simulink subsystem "ButtonStatesRF"  (R2023b) (continued)
== stateflow chart ButtonStatesRF/ButtonStates/buttonFlowChart == (continued)
                (junction 6907) -> 6908
                QS_DOWN_SubMenu -> 6908 [order 2] : EXIT
                QS_DOWN_SubMenu -> (junction 6907) [order 1] : SET
                    | {quickSelectorDown = currentState;
                    | BLU_FnSetQuickSelectorDOWN(quickSelectorDown);}
                6909 -> QS_DOWN_SubMenu
              QuickSelectorUP -> 6783 [order 1] : SET
              6784 -> QuickSelectorSubMenu1
              6785 -> QuickSelectorUP
              QuickSelectorSubMenu1 -> 6786 : EXIT
              QuickSelectorDOWN -> 6787 [order 1] : SET
              6788 -> QuickSelectorDOWN
            state ConnectivityMenu
              (history)
              state ConnectivitySubMenu
                (history)
                state ConnectivitySubMenu
                  state Bluetooth
                    en:
                    currentState = eStateName.CONNECTIVITY_BLUETOOTH;
                  state PhonePairing
                    en:
                    currentState = eStateName.CONNECTIVITY_PHONEPAIRING;
                  state HeadsetPairing
                    en:
                    currentState = eStateName.CONNECTIVITY_HEADSETPAIRING;
                  state HeadsetType
                    en:
                    currentState = eStateName.CONNECTIVITY_HEADSETTYPE;
                  (default) -> (junction 7044)
                  (junction 7044) -> Bluetooth
                  (junction 7051) -> (junction 7044)
                  Bluetooth -> (junction 7045) [order 3] : UP
                  (junction 7045) -> (junction 7050) : [CCU_BT_Status]
                  Bluetooth -> (junction 7046) [order 1] : DOWN
                  PhonePairing -> Bluetooth [order 2] : UP
                  (junction 7046) -> PhonePairing : [CCU_BT_Status]
                  (junction 7049) -> (junction 7046) [order 2]
                  PhonePairing -> (junction 7047) [order 1] : DOWN
                  (junction 7047) -> (junction 7048)
                  HeadsetPairing -> PhonePairing [order 1] : UP
                  (junction 7048) -> HeadsetPairing [order 1] : [CCU_Corded_Headset == 0]
                  (junction 7048) -> (junction 7050) [order 2]
                  (junction 7049) -> HeadsetPairing [order 1] : [CCU_Corded_Headset == 0]
                  HeadsetType -> (junction 7049) [order 1] : UP
                  HeadsetPairing -> HeadsetType [order 2] : DOWN
                  (junction 7050) -> HeadsetType
                  HeadsetType -> (junction 7051) [order 3] : DOWN
                state BLUETOOTH_ONOFF
                  (history)
                  state BT_Sel
                    state BLUETOOTH_ON
                      en:
                      currentState = eStateName.CONNECTIVITY_BLUETOOTH_ON;
                    state BLUETOOTH_OFF
                      en:
                      currentState = eStateName.CONNECTIVITY_BLUETOOTH_OFF ;
                    (junction 7084) -> BLUETOOTH_ON [order 1] : [CCU_BT_Status]
                    BLUETOOTH_ON -> BLUETOOTH_OFF : DOWN || UP
                    BLUETOOTH_OFF -> BLUETOOTH_ON : DOWN || UP
                    (default) -> (junction 7084)
                    (junction 7084) -> BLUETOOTH_OFF [order 2]
                  BT_Sel -> (junction 7076) [order 2] : SET
                  (junction 7076) -> 7077 : {BLU_FnConnBTSwitch(currentState);}
                  BT_Sel -> 7077 [order 1] : EXIT
                  7078 -> (junction 7084)
                state PhoneMenu
                  (history)
                  state PhonePairinSubMenu
                    state NEW_PAIRING
                      en:
                      currentState = eStateName.PHONE_PAIRING_NEW;
                    state DELETE_PAIRING
                      en:
                      currentState = eStateName.PHONE_PAIRING_DELETE ;
                    (junction 7112) -> NEW_PAIRING [order 2]
                    NEW_PAIRING -> (junction 7113) [order 2] : DOWN || UP
                    NEW_PAIRING -> DELETE_PAIRING [order 3] : [hasChangedTo(btPhonePrngScs,true)]
                    DELETE_PAIRING -> NEW_PAIRING [order 3] : [hasChangedTo(btPhonePrngScs,false)]
                    (junction 7111) -> (junction 7112)
                    (junction 7113) -> (junction 7112)
                    (default) -> (junction 7111)
                    (junction 7112) -> DELETE_PAIRING [order 1] : [btPhonePrngScs]
                    DELETE_PAIRING -> (junction 7113) [order 1] : DOWN || UP
                  state PhoneDeviceSearch
                    (history)
                    state BT_PHONE_STRT_SEARCHING
                      en:
                      currentState=eStateName.BT_PHONE_STRT_SEARCHING;
                    state BT_PHONE_FOUND
                      en:
                      currentState=eStateName.BT_PHONE_DVC_LIST;
                    state BT_PHONE_ALREADY_PAIRED
                      en:
                      currentState=eStateName.BT_PHONE_ALREADY_PAIRED;
                    state BT_PHONE_NOT_FOUND [commented out]
                      en:
                      currentState=eStateName.BT_PHONE_NOT_FOUND;
                    state BT_PHONE_PAIRING
                      en:
                      currentState=eStateName.BT_PHONE_PAIRING_INPROGRESS;
                    state BT_PHONE_CHECK_PASSKEY
                      en:
                      currentState=eStateName.BT_PHONE_CHECK_PASSKEY;
                    state BT_PHONE_PAIRING_FAILED
                      en:
                      currentState=eStateName.BT_PHONE_PAIRING_FAILED;
                    state BT_PHONE_PAIR_SUCCESS
                      en:
                      currentState=eStateName.BT_PHONE_PAIR_SUCCESS;
                    7146 -> Flow.ButtonTriggeredMenu.Menu.MainSubMenu.SettingsSubMenu.ConnectivityMenu.ConnectivitySubMenu.PhoneMenu.PhoneDeviceSearch.BT_PHONE_STRT_SEARCHING
                    (default) -> Flow.ButtonTriggeredMenu.Menu.MainSubMenu.SettingsSubMenu.ConnectivityMenu.ConnectivitySubMenu.PhoneMenu.PhoneDeviceSearch.BT_PHONE_STRT_SEARCHING
                    Flow.ButtonTriggeredMenu.Menu.MainSubMenu.SettingsSubMenu.ConnectivityMenu.ConnectivitySubMenu.PhoneMenu.PhoneDeviceSearch.BT_PHONE_FOUND -> (junction 7127) [order 1] : UP
                    (junction 7127) -> (junction 7128) : {BLU_FnBTPhoneDvcUp();}
                    Flow.ButtonTriggeredMenu.Menu.MainSubMenu.SettingsSubMenu.ConnectivityMenu.ConnectivitySubMenu.PhoneMenu.PhoneDeviceSearch.BT_PHONE_STRT_SEARCHING -> Flow.ButtonTriggeredMenu.Menu.MainSubMenu.SettingsSubMenu.ConnectivityMenu.ConnectivitySubMenu.PhoneMenu.PhoneDeviceSearch.BT_PHONE_FOUND [order 1] : [hasChangedTo(btPhoneFound,true)]
                    (junction 7128) -> Flow.ButtonTriggeredMenu.Menu.MainSubMenu.SettingsSubMenu.ConnectivityMenu.ConnectivitySubMenu.PhoneMenu.PhoneDeviceSearch.BT_PHONE_FOUND
                    (junction 7129) -> (junction 7128) : {BLU_FnBTPhoneDvcDwn();}
                    Flow.ButtonTriggeredMenu.Menu.MainSubMenu.SettingsSubMenu.ConnectivityMenu.ConnectivitySubMenu.PhoneMenu.PhoneDeviceSearch.BT_PHONE_STRT_SEARCHING -> (junction 7130) [order 3] : after(BLU_BTSearchDur_Tms,msec)
                    Flow.ButtonTriggeredMenu.Menu.MainSubMenu.SettingsSubMenu.ConnectivityMenu.ConnectivitySubMenu.PhoneMenu.PhoneDeviceSearch.BT_PHONE_STRT_SEARCHING -> (junction 7131) [order 2] : EXIT
                    Flow.ButtonTriggeredMenu.Menu.MainSubMenu.SettingsSubMenu.ConnectivityMenu.ConnectivitySubMenu.PhoneMenu.PhoneDeviceSearch.BT_PHONE_FOUND -> (junction 7129) [order 2] : DOWN
                    (junction 7130) -> (junction 7131)
                    (junction 7131) -> (junction 7132)
                    Flow.ButtonTriggeredMenu.Menu.MainSubMenu.SettingsSubMenu.ConnectivityMenu.ConnectivitySubMenu.PhoneMenu.PhoneDeviceSearch.BT_PHONE_FOUND -> (junction 7132) [order 3] : EXIT
                    Flow.ButtonTriggeredMenu.Menu.MainSubMenu.SettingsSubMenu.ConnectivityMenu.ConnectivitySubMenu.PhoneMenu.PhoneDeviceSearch.BT_PHONE_FOUND -> (junction 7133) [order 4] : SET
                    (junction 7132) -> (junction 7135)
                    (junction 7133) -> (junction 7134)
                    (junction 7134) -> Flow.ButtonTriggeredMenu.Menu.MainSubMenu.SettingsSubMenu.ConnectivityMenu.ConnectivitySubMenu.PhoneMenu.PhoneDeviceSearch.BT_PHONE_ALREADY_PAIRED [order 1] : %[phonePaired]
                        | [btPhonePrngScs]
                    (junction 7134) -> (junction 7136) [order 2]
                    Flow.ButtonTriggeredMenu.Menu.MainSubMenu.SettingsSubMenu.ConnectivityMenu.ConnectivitySubMenu.PhoneMenu.PhoneDeviceSearch.BT_PHONE_NOT_FOUND -> (junction 7135) [commented out] : EXIT
                    Flow.ButtonTriggeredMenu.Menu.MainSubMenu.SettingsSubMenu.ConnectivityMenu.ConnectivitySubMenu.PhoneMenu.PhoneDeviceSearch.BT_PHONE_ALREADY_PAIRED -> (junction 7135) [order 2] : EXIT
                    (junction 7135) -> (junction 7140)
                    Flow.ButtonTriggeredMenu.Menu.MainSubMenu.SettingsSubMenu.ConnectivityMenu.ConnectivitySubMenu.PhoneMenu.PhoneDeviceSearch.BT_PHONE_ALREADY_PAIRED -> (junction 7136) [order 1] : SET
                    (junction 7136) -> Flow.ButtonTriggeredMenu.Menu.MainSubMenu.SettingsSubMenu.ConnectivityMenu.ConnectivitySubMenu.PhoneMenu.PhoneDeviceSearch.BT_PHONE_PAIRING : {BLU_FnBTPhonePairReq();}
                    (junction 7138) -> (junction 7137)
                    (junction 7137) -> (junction 7139)
                    Flow.ButtonTriggeredMenu.Menu.MainSubMenu.SettingsSubMenu.ConnectivityMenu.ConnectivitySubMenu.PhoneMenu.PhoneDeviceSearch.BT_PHONE_CHECK_PASSKEY -> (junction 7138) [order 2] : [hasChangedTo(btPhonePairingError,true)]
                    Flow.ButtonTriggeredMenu.Menu.MainSubMenu.SettingsSubMenu.ConnectivityMenu.ConnectivitySubMenu.PhoneMenu.PhoneDeviceSearch.BT_PHONE_CHECK_PASSKEY -> (junction 7139) [order 1] : EXIT
                    Flow.ButtonTriggeredMenu.Menu.MainSubMenu.SettingsSubMenu.ConnectivityMenu.ConnectivitySubMenu.PhoneMenu.PhoneDeviceSearch.BT_PHONE_PAIRING -> Flow.ButtonTriggeredMenu.Menu.MainSubMenu.SettingsSubMenu.ConnectivityMenu.ConnectivitySubMenu.PhoneMenu.PhoneDeviceSearch.BT_PHONE_CHECK_PASSKEY [order 3] : [hasChangedTo(btPhonePassKeyRcvd,true)]
                    (junction 7139) -> Flow.ButtonTriggeredMenu.Menu.MainSubMenu.SettingsSubMenu.ConnectivityMenu.ConnectivitySubMenu.PhoneMenu.PhoneDeviceSearch.BT_PHONE_PAIRING_FAILED
                    Flow.ButtonTriggeredMenu.Menu.MainSubMenu.SettingsSubMenu.ConnectivityMenu.ConnectivitySubMenu.PhoneMenu.PhoneDeviceSearch.BT_PHONE_CHECK_PASSKEY -> (junction 7140) [order 4] : [after(25,sec)]
                    Flow.ButtonTriggeredMenu.Menu.MainSubMenu.SettingsSubMenu.ConnectivityMenu.ConnectivitySubMenu.PhoneMenu.PhoneDeviceSearch.BT_PHONE_CHECK_PASSKEY -> Flow.ButtonTriggeredMenu.Menu.MainSubMenu.SettingsSubMenu.ConnectivityMenu.ConnectivitySubMenu.PhoneMenu.PhoneDeviceSearch.BT_PHONE_PAIRING [order 3] : SET
                        | {BLU_FnBTPhonePassKeyChkd();}
                    (junction 7140) -> (junction 7141)
                    Flow.ButtonTriggeredMenu.Menu.MainSubMenu.SettingsSubMenu.ConnectivityMenu.ConnectivitySubMenu.PhoneMenu.PhoneDeviceSearch.BT_PHONE_PAIRING -> (junction 7142) [order 4] : EXIT
                    Flow.ButtonTriggeredMenu.Menu.MainSubMenu.SettingsSubMenu.ConnectivityMenu.ConnectivitySubMenu.PhoneMenu.PhoneDeviceSearch.BT_PHONE_PAIRING -> (junction 7143) [order 1] : [hasChangedTo(btPhonePairingError,true)]
                    Flow.ButtonTriggeredMenu.Menu.MainSubMenu.SettingsSubMenu.ConnectivityMenu.ConnectivitySubMenu.PhoneMenu.PhoneDeviceSearch.BT_PHONE_PAIRING -> (junction 7144) [order 2] : [hasChangedTo(btPhonePrngScs,true)]
                    Flow.ButtonTriggeredMenu.Menu.MainSubMenu.SettingsSubMenu.ConnectivityMenu.ConnectivitySubMenu.PhoneMenu.PhoneDeviceSearch.BT_PHONE_PAIRING_FAILED -> (junction 7141) : [after(3,sec)]
                        | {phonePaired=false;}
                    (junction 7142) -> (junction 7141)
                    (junction 7143) -> (junction 7142)
                    (junction 7141) -> (junction 7145)
                    (junction 7145) -> 7147
                    Flow.ButtonTriggeredMenu.Menu.MainSubMenu.SettingsSubMenu.ConnectivityMenu.ConnectivitySubMenu.PhoneMenu.PhoneDeviceSearch.BT_PHONE_PAIR_SUCCESS -> (junction 7145) : after(1,sec)
                    (junction 7144) -> Flow.ButtonTriggeredMenu.Menu.MainSubMenu.SettingsSubMenu.ConnectivityMenu.ConnectivitySubMenu.PhoneMenu.PhoneDeviceSearch.BT_PHONE_PAIR_SUCCESS : {phonePaired=true;}
                  state PhoneBTDvcDelete
                    (history)
                    state BT_PHONE_DEL_PAIRING_WAIT
                      en:
                      currentState=eStateName.BT_PHONE_DEL_PAIRING_WAIT;
                      BLU_FnBTPhoneDvcDel();
                    7202 -> (junction 7201)
                    (junction 7200) -> 7203
                    BT_PHONE_DEL_PAIRING_WAIT -> (junction 7200) [order 1] : EXIT
                    BT_PHONE_DEL_PAIRING_WAIT -> 7203 [order 2] : [hasChangedTo(btPhoneDelErr,true)]
                    (default) -> (junction 7201)
                    (junction 7201) -> BT_PHONE_DEL_PAIRING_WAIT
                    BT_PHONE_DEL_PAIRING_WAIT -> 7204 [order 3] : [hasChangedTo(btPhonePrngScs, false)]
                  NEW_PAIRING -> 7095 [order 1] : SET
                  7096 -> PhonePairinSubMenu
                  (junction 7094) -> NEW_PAIRING
                  7098 -> (junction 7094)
                  7097 -> (junction 7111)
                  DELETE_PAIRING -> 7099 [order 2] : SET
                  PhonePairinSubMenu -> 7100 : EXIT
                  7101 -> DELETE_PAIRING
                state BT_HeadsetMenu
                  (history)
                  state HeadsetPairingSubMenu
                    state HEADSET_PAIRING_NEW
                      en:
                      currentState = eStateName.HEADSET_PAIRING_NEW;
                    state HEADSET_PAIRING_DELETE
                      en:
                      currentState = eStateName.HEADSET_PAIRING_DELETE;
                    (junction 7232) -> HEADSET_PAIRING_NEW [order 2]
                    HEADSET_PAIRING_NEW -> (junction 7233) [order 1] : DOWN || UP
                    HEADSET_PAIRING_NEW -> HEADSET_PAIRING_DELETE [order 3] : [hasChangedTo(btHdstPrngScs,true)]
                    (junction 7234) -> HEADSET_PAIRING_NEW
                    (default) -> (junction 7231)
                    (junction 7231) -> (junction 7232)
                    (junction 7233) -> (junction 7232)
                    (junction 7232) -> HEADSET_PAIRING_DELETE [order 1] : [btHdstPrngScs]
                    HEADSET_PAIRING_DELETE -> (junction 7233) [order 2] : DOWN || UP
                    HEADSET_PAIRING_DELETE -> (junction 7234) [order 1] : [hasChangedTo(btHdstPrngScs,false)]
                  state HeadsetDeviceSearch
                    (history)
                    state BT_HEADSET_FOUND
                      en:
                      currentState=eStateName.BT_HEADSET_DVC_LIST;
                    state BT_HEADSET_STRT_SEARCHING
                      en:
                      currentState=eStateName.BT_HEADSET_STRT_SEARCHING;
                    state PhoneDeviceSearch [commented out]
                      state BT_PHONE_STRT_SEARCHING [commented out]
                        en:
                        currentState=eStateName.BT_PHONE_STRT_SEARCHING;
                      state BT_PHONE_FOUND [commented out]
                        en:
                        currentState=eStateName.BT_PHONE_DVC_LIST;
                      state BT_PHONE_ALREADY_PAIRED [commented out]
                        en:
                        currentState=eStateName.BT_PHONE_ALREADY_PAIRED;
                      state BT_PHONE_NOT_FOUND [commented out]
                        en:
                        currentState=eStateName.BT_PHONE_NOT_FOUND;
                      state BT_PHONE_PAIRING [commented out]
                        en:
                        currentState=eStateName.BT_PHONE_PAIRING_INPROGRESS;
                      state BT_PHONE_CHECK_PASSKEY [commented out]
                        en:
                        currentState=eStateName.BT_PHONE_CHECK_PASSKEY;
                      state BT_PHONE_PAIRING_FAILED [commented out]
                        en:
                        currentState=eStateName.BT_PHONE_PAIRING_FAILED;
                      state BT_PHONE_PAIR_SUCCESS [commented out]
                        en:
                        currentState=eStateName.BT_PHONE_PAIR_SUCCESS;
                      (default) -> Flow.ButtonTriggeredMenu.Menu.MainSubMenu.SettingsSubMenu.ConnectivityMenu.ConnectivitySubMenu.BT_HeadsetMenu.HeadsetDeviceSearch.PhoneDeviceSearch.BT_PHONE_STRT_SEARCHING [commented out]
                      Flow.ButtonTriggeredMenu.Menu.MainSubMenu.SettingsSubMenu.ConnectivityMenu.ConnectivitySubMenu.BT_HeadsetMenu.HeadsetDeviceSearch.PhoneDeviceSearch.BT_PHONE_FOUND -> (junction 7303) [commented out] : UP
                      (junction 7303) -> (junction 7304) [commented out] : {BLU_FnBTPhoneDvcUp();}
                      Flow.ButtonTriggeredMenu.Menu.MainSubMenu.SettingsSubMenu.ConnectivityMenu.ConnectivitySubMenu.BT_HeadsetMenu.HeadsetDeviceSearch.PhoneDeviceSearch.BT_PHONE_STRT_SEARCHING -> Flow.ButtonTriggeredMenu.Menu.MainSubMenu.SettingsSubMenu.ConnectivityMenu.ConnectivitySubMenu.BT_HeadsetMenu.HeadsetDeviceSearch.PhoneDeviceSearch.BT_PHONE_FOUND [commented out] : [hasChangedTo(btPhoneFound,true)]
                      (junction 7304) -> Flow.ButtonTriggeredMenu.Menu.MainSubMenu.SettingsSubMenu.ConnectivityMenu.ConnectivitySubMenu.BT_HeadsetMenu.HeadsetDeviceSearch.PhoneDeviceSearch.BT_PHONE_FOUND [commented out]
                      (junction 7305) -> (junction 7304) [commented out] : {BLU_FnBTPhoneDvcDwn();}
                      Flow.ButtonTriggeredMenu.Menu.MainSubMenu.SettingsSubMenu.ConnectivityMenu.ConnectivitySubMenu.BT_HeadsetMenu.HeadsetDeviceSearch.PhoneDeviceSearch.BT_PHONE_STRT_SEARCHING -> (junction 7306) [commented out] : after(BLU_BTSearchDur_Tms,msec)
                      Flow.ButtonTriggeredMenu.Menu.MainSubMenu.SettingsSubMenu.ConnectivityMenu.ConnectivitySubMenu.BT_HeadsetMenu.HeadsetDeviceSearch.PhoneDeviceSearch.BT_PHONE_FOUND -> (junction 7305) [commented out] : DOWN
                      (junction 7306) -> (junction 7307) [commented out]
                      Flow.ButtonTriggeredMenu.Menu.MainSubMenu.SettingsSubMenu.ConnectivityMenu.ConnectivitySubMenu.BT_HeadsetMenu.HeadsetDeviceSearch.PhoneDeviceSearch.BT_PHONE_FOUND -> (junction 7307) [commented out] : EXIT
                      Flow.ButtonTriggeredMenu.Menu.MainSubMenu.SettingsSubMenu.ConnectivityMenu.ConnectivitySubMenu.BT_HeadsetMenu.HeadsetDeviceSearch.PhoneDeviceSearch.BT_PHONE_FOUND -> (junction 7308) [commented out] : SET
                      (junction 7307) -> (junction 7310) [commented out]
                      (junction 7308) -> (junction 7309) [commented out]
                      (junction 7309) -> Flow.ButtonTriggeredMenu.Menu.MainSubMenu.SettingsSubMenu.ConnectivityMenu.ConnectivitySubMenu.BT_HeadsetMenu.HeadsetDeviceSearch.PhoneDeviceSearch.BT_PHONE_ALREADY_PAIRED [commented out] : [phonePaired]
                      (junction 7309) -> (junction 7311) [commented out]
                      Flow.ButtonTriggeredMenu.Menu.MainSubMenu.SettingsSubMenu.ConnectivityMenu.ConnectivitySubMenu.BT_HeadsetMenu.HeadsetDeviceSearch.PhoneDeviceSearch.BT_PHONE_NOT_FOUND -> (junction 7310) [commented out] : EXIT
                      Flow.ButtonTriggeredMenu.Menu.MainSubMenu.SettingsSubMenu.ConnectivityMenu.ConnectivitySubMenu.BT_HeadsetMenu.HeadsetDeviceSearch.PhoneDeviceSearch.BT_PHONE_ALREADY_PAIRED -> (junction 7310) [commented out] : EXIT
                      (junction 7310) -> (junction 7313) [commented out]
                      Flow.ButtonTriggeredMenu.Menu.MainSubMenu.SettingsSubMenu.ConnectivityMenu.ConnectivitySubMenu.BT_HeadsetMenu.HeadsetDeviceSearch.PhoneDeviceSearch.BT_PHONE_ALREADY_PAIRED -> (junction 7311) [commented out] : SET
                      (junction 7311) -> Flow.ButtonTriggeredMenu.Menu.MainSubMenu.SettingsSubMenu.ConnectivityMenu.ConnectivitySubMenu.BT_HeadsetMenu.HeadsetDeviceSearch.PhoneDeviceSearch.BT_PHONE_PAIRING [commented out] : {BLU_FnBTPhonePairReq();}
                      Flow.ButtonTriggeredMenu.Menu.MainSubMenu.SettingsSubMenu.ConnectivityMenu.ConnectivitySubMenu.BT_HeadsetMenu.HeadsetDeviceSearch.PhoneDeviceSearch.BT_PHONE_PAIRING -> Flow.ButtonTriggeredMenu.Menu.MainSubMenu.SettingsSubMenu.ConnectivityMenu.ConnectivitySubMenu.BT_HeadsetMenu.HeadsetDeviceSearch.PhoneDeviceSearch.BT_PHONE_CHECK_PASSKEY [commented out] : [hasChangedTo(btPhonePassKeyRcvd,true)]
                      Flow.ButtonTriggeredMenu.Menu.MainSubMenu.SettingsSubMenu.ConnectivityMenu.ConnectivitySubMenu.BT_HeadsetMenu.HeadsetDeviceSearch.PhoneDeviceSearch.BT_PHONE_CHECK_PASSKEY -> Flow.ButtonTriggeredMenu.Menu.MainSubMenu.SettingsSubMenu.ConnectivityMenu.ConnectivitySubMenu.BT_HeadsetMenu.HeadsetDeviceSearch.PhoneDeviceSearch.BT_PHONE_PAIRING [commented out] : SET
                          | {BLU_FnBTPhonePassKeyChkd();}
                      Flow.ButtonTriggeredMenu.Menu.MainSubMenu.SettingsSubMenu.ConnectivityMenu.ConnectivitySubMenu.BT_HeadsetMenu.HeadsetDeviceSearch.PhoneDeviceSearch.BT_PHONE_CHECK_PASSKEY -> (junction 7314) [commented out] : EXIT
                      Flow.ButtonTriggeredMenu.Menu.MainSubMenu.SettingsSubMenu.ConnectivityMenu.ConnectivitySubMenu.BT_HeadsetMenu.HeadsetDeviceSearch.PhoneDeviceSearch.BT_PHONE_PAIRING -> (junction 7312) [commented out] : EXIT
                      Flow.ButtonTriggeredMenu.Menu.MainSubMenu.SettingsSubMenu.ConnectivityMenu.ConnectivitySubMenu.BT_HeadsetMenu.HeadsetDeviceSearch.PhoneDeviceSearch.BT_PHONE_PAIRING -> (junction 7315) [commented out] : [hasChangedTo(btPhonePairingError,true)]
                      Flow.ButtonTriggeredMenu.Menu.MainSubMenu.SettingsSubMenu.ConnectivityMenu.ConnectivitySubMenu.BT_HeadsetMenu.HeadsetDeviceSearch.PhoneDeviceSearch.BT_PHONE_PAIRING -> (junction 7316) [commented out] : [hasChangedTo(btPhonePairingScs,true)]
                      Flow.ButtonTriggeredMenu.Menu.MainSubMenu.SettingsSubMenu.ConnectivityMenu.ConnectivitySubMenu.BT_HeadsetMenu.HeadsetDeviceSearch.PhoneDeviceSearch.BT_PHONE_PAIRING_FAILED -> (junction 7313) [commented out] : SET || UP||DOWN || EXIT
                      (junction 7314) -> (junction 7313) [commented out]
                      (junction 7312) -> (junction 7314) [commented out]
                      (junction 7315) -> (junction 7312) [commented out]
                      (junction 7313) -> (junction 7317) [commented out]
                      (junction 7317) -> 7318 [commented out]
                      Flow.ButtonTriggeredMenu.Menu.MainSubMenu.SettingsSubMenu.ConnectivityMenu.ConnectivitySubMenu.BT_HeadsetMenu.HeadsetDeviceSearch.PhoneDeviceSearch.BT_PHONE_PAIR_SUCCESS -> (junction 7317) [commented out] : after(3,sec)
                      (junction 7316) -> Flow.ButtonTriggeredMenu.Menu.MainSubMenu.SettingsSubMenu.ConnectivityMenu.ConnectivitySubMenu.BT_HeadsetMenu.HeadsetDeviceSearch.PhoneDeviceSearch.BT_PHONE_PAIR_SUCCESS [commented out] : {phonePaired=true;}
                    state BT_HEADSET_NOT_FOUND [commented out]
                      en:
                      currentState=eStateName.BT_HEADSET_NOT_FOUND;
                    state BT_HEADSET_ALREADY_PAIRED
                      en:
                      currentState=eStateName.BT_HEADSET_ALREADY_PAIRED;
                    state BT_HEADSET_PAIRING_INPROGRESS
                      en:
                      currentState=eStateName.BT_HEADSET_PAIRING_INPROGRESS;
                    state BT_HEADSET_PAIRING_FAILED
                      en:
                      currentState=eStateName.BT_HEADSET_PAIRING_FAILED;
                    state BT_HEADSET_PAIR_SUCCESS
                      en:
                      currentState=eStateName.BT_HEADSET_PAIR_SUCCESS;
                    7264 -> BT_HEADSET_STRT_SEARCHING
                    (default) -> BT_HEADSET_STRT_SEARCHING
                    BT_HEADSET_FOUND -> (junction 7249) [order 2] : UP
                    (junction 7249) -> (junction 7250) : {BLU_FnBTHeadsetDvcUp();}
                    (junction 7250) -> BT_HEADSET_FOUND
                    BT_HEADSET_STRT_SEARCHING -> BT_HEADSET_FOUND [order 1] : [hasChangedTo(btHeadsetFound,true)]
                        | {headsetPaired = btHdstPrngScs;}
                    (junction 7252) -> (junction 7250) : {BLU_FnBTHeadsetDvcDwn();}
                    BT_HEADSET_STRT_SEARCHING -> (junction 7251) [order 3] : after(BLU_BTSearchDur_Tms,msec)
                    BT_HEADSET_FOUND -> (junction 7251) [order 4] : EXIT
                    BT_HEADSET_FOUND -> (junction 7252) [order 3] : DOWN
                    (junction 7251) -> (junction 7255)
                    BT_HEADSET_STRT_SEARCHING -> (junction 7254) [order 2] : EXIT
                    BT_HEADSET_FOUND -> (junction 7253) [order 1] : SET
                    (junction 7253) -> (junction 7256)
                    (junction 7254) -> (junction 7255)
                    (junction 7255) -> (junction 7257)
                    (junction 7256) -> BT_HEADSET_ALREADY_PAIRED [order 1] : [headsetPaired]
                    (junction 7256) -> (junction 7258) [order 2]
                    BT_HEADSET_NOT_FOUND -> (junction 7257) [commented out] : EXIT
                    BT_HEADSET_ALREADY_PAIRED -> (junction 7257) [order 1] : EXIT
                    (junction 7257) -> (junction 7259)
                    BT_HEADSET_ALREADY_PAIRED -> (junction 7258) [order 2] : SET
                    (junction 7258) -> BT_HEADSET_PAIRING_INPROGRESS : {BLU_FnBTHeadsetPairReq();}
                    BT_HEADSET_PAIRING_INPROGRESS -> BT_HEADSET_PAIRING_FAILED [order 2] : [hasChangedTo(btHeadsetPairingError,true)]
                    BT_HEADSET_PAIRING_FAILED -> (junction 7259) : SET || UP||DOWN || EXIT
                    (junction 7259) -> (junction 7260)
                    BT_HEADSET_PAIRING_INPROGRESS -> (junction 7261) [order 1]
                    BT_HEADSET_PAIRING_INPROGRESS -> (junction 7263) [order 3] : EXIT
                    (junction 7260) -> 7265
                    BT_HEADSET_PAIR_SUCCESS -> (junction 7260) : after(3,sec)
                        | {headsetPaired = btHdstPrngScs;}
                    (junction 7261) -> BT_HEADSET_PAIR_SUCCESS : [hasChangedTo(btHdstPrngScs,true)]
                    (junction 7262) -> (junction 7260)
                    (junction 7263) -> (junction 7262)
                  state HeadsetBTDvcDelete
                    (history)
                    state BT_HEADSET_DEL_PAIRING_WAIT
                      en:
                      currentState=eStateName.BT_HEADSET_DEL_PAIRING_WAIT;
                      BLU_FnBTHeadsetDvcDel();
                    (junction 7367) -> 7371
                    7370 -> (junction 7369)
                    BT_HEADSET_DEL_PAIRING_WAIT -> (junction 7367) [order 1] : EXIT
                    BT_HEADSET_DEL_PAIRING_WAIT -> 7371 [order 2] : [hasChangedTo(btHdstDelErr,true)]
                        | {headsetPaired = btHdstPrngScs;}
                    (default) -> (junction 7369)
                    (junction 7369) -> BT_HEADSET_DEL_PAIRING_WAIT
                    BT_HEADSET_DEL_PAIRING_WAIT -> 7372 [order 3] : [hasChangedTo(btHdstPrngScs,false)]
                  HEADSET_PAIRING_NEW -> 7215 [order 2] : SET
                  7216 -> HeadsetPairingSubMenu
                  7217 -> (junction 7231)
                  (junction 7214) -> (junction 7234)
                  7218 -> (junction 7214)
                  HeadsetPairingSubMenu -> 7219 : EXIT
                  HEADSET_PAIRING_DELETE -> 7220 [order 3] : SET
                  7221 -> HEADSET_PAIRING_DELETE
                state HeadsetTypeMenu
                  state HeadsetSel
                    (history)
                    state HEADSET_CORDED
                      en:
                      currentState = eStateName.HEADSETTYPE_CORDED;
                    state HEADSET_BLUETOOTH
                      en:
                      currentState = eStateName.HEADSETTYPE_BLUETOOTH ;
                    (junction 7394) -> HEADSET_CORDED [order 1] : [CCU_Corded_Headset == 1]
                    (junction 7394) -> (junction 7395) [order 2]
                    HEADSET_CORDED -> HEADSET_BLUETOOTH [order 1] : DOWN || UP
                    HEADSET_BLUETOOTH -> HEADSET_CORDED [order 1] : DOWN || UP
                    (junction 7395) -> HEADSET_BLUETOOTH
                  7384 -> (junction 7394)
                  HEADSET_CORDED -> (junction 7382) [order 2] : SET
                  (junction 7382) -> 7385 : {BLU_FnHdstSelCord();}
                  HeadsetSel -> 7385 : EXIT
                  (junction 7383) -> 7385 : {BLU_FnHdstSelBT();}
                  HEADSET_BLUETOOTH -> (junction 7383) [order 2] : SET
                Bluetooth -> 7026 [order 2] : SET
                7027 -> Bluetooth
                (default) -> Flow.ButtonTriggeredMenu.Menu.MainSubMenu.SettingsSubMenu.ConnectivityMenu.ConnectivitySubMenu.ConnectivitySubMenu
                PhonePairing -> 7028 [order 3] : SET
                7029 -> PhonePairing
                HeadsetPairing -> 7030 [order 3] : SET
                7031 -> HeadsetPairing
                HeadsetType -> 7032 [order 2] : SET
                7033 -> HeadsetType
              state AppReqMenu
                en:
                popUpState = eStateName.POPUP_REG_APP_REPLY;
              state CnfrmAppReqWait
              7014 -> (junction 7044)
              Flow.ButtonTriggeredMenu.Menu.MainSubMenu.SettingsSubMenu.ConnectivityMenu.ConnectivitySubMenu.ConnectivitySubMenu -> 7015 : EXIT
              Flow.ButtonTriggeredMenu.Menu.MainSubMenu.SettingsSubMenu.ConnectivityMenu.ConnectivitySubMenu -> AppReqMenu : [hasChangedTo(rgstrAppReply,0)]
              CnfrmAppReqWait -> Flow.ButtonTriggeredMenu.Menu.MainSubMenu.SettingsSubMenu.ConnectivityMenu.ConnectivitySubMenu : after(1,sec)
              AppReqMenu -> (junction 7012) [order 1] : SET
                  | {cnfrmAppReg = 0;}
              (junction 7012) -> CnfrmAppReqWait : {BLU_FnCnctvtyCnfrmAppReg(cnfrmAppReg);}
              (junction 7013) -> (junction 7012)
              AppReqMenu -> (junction 7013) [order 2] : EXIT
                  | {cnfrmAppReg = 15}
            state QuickShifterMenu
              state QuickShifterSubMenu
                (history)
                state QuickShifterOFF
                  en:
                  currentState = eStateName.QUICKSHIFTER_OFF;
                state QuickShifterON
                  en:
                  currentState = eStateName.QUICKSHIFTER_ON;
                (default) -> QuickShifterOFF
                QuickShifterOFF -> QuickShifterON : DOWN || UP
                QuickShifterON -> QuickShifterOFF : UP || DOWN
              7407 -> QuickShifterSubMenu
              QuickShifterSubMenu -> (junction 7406) [order 2] : SET
                  | {BLU_FnQuickShifterSwitch(currentState);}
              (junction 7406) -> 7408
              QuickShifterSubMenu -> 7408 [order 1] : EXIT
            state ShiftLightSubMenu
              (history)
              state OdoLessKm
                en:
                currentState = eStateName.ODO_MSG_KM;
              state ShiftLightMenu1
                (history)
                state SL_RPM1
                  en:
                  currentState = eStateName.SL_RPM1;
                state SL_RPM2
                  en:
                  currentState = eStateName.SL_RPM2;
                state ShiftLight
                  en:
                  currentState = eStateName.SHIFT_LIGHT_ON_OFF;
                (junction 7467) -> SL_RPM1 : [ShiftLightStatus == 1]
                (junction 7470) -> (junction 7467) [order 2] : DOWN
                SL_RPM1 -> SL_RPM2 [order 2] : DOWN
                SL_RPM2 -> SL_RPM1 [order 2] : UP
                (junction 7469) -> SL_RPM2 : [ShiftLightStatus == 1]
                SL_RPM2 -> Flow.ButtonTriggeredMenu.Menu.MainSubMenu.SettingsSubMenu.ShiftLightSubMenu.ShiftLightMenu1.ShiftLight [order 3] : DOWN
                Flow.ButtonTriggeredMenu.Menu.MainSubMenu.SettingsSubMenu.ShiftLightSubMenu.ShiftLightMenu1.ShiftLight -> (junction 7469) [order 1] : UP
                Flow.ButtonTriggeredMenu.Menu.MainSubMenu.SettingsSubMenu.ShiftLightSubMenu.ShiftLightMenu1.ShiftLight -> (junction 7470) [order 2]
              state RPM1menu
                state RPM1menu1
                  en:
                  currentState = eStateName.SL_SET_RPM1;
                7495 -> (junction 7483)
                (default) -> (junction 7483)
                (junction 7483) -> (junction 7484) [order 1] : [rpm1 >= 5000]
                (junction 7483) -> (junction 7485) [order 2]
                (junction 7484) -> Flow.ButtonTriggeredMenu.Menu.MainSubMenu.SettingsSubMenu.ShiftLightSubMenu.RPM1menu.RPM1menu1
                (junction 7485) -> Flow.ButtonTriggeredMenu.Menu.MainSubMenu.SettingsSubMenu.ShiftLightSubMenu.RPM1menu.RPM1menu1 : {rpm1=5000;}
                Flow.ButtonTriggeredMenu.Menu.MainSubMenu.SettingsSubMenu.ShiftLightSubMenu.RPM1menu.RPM1menu1 -> (junction 7486) [order 1] : UP
                (junction 7486) -> (junction 7487) [order 1] : [rpm1<11000 && rpm1 >= 5000]
                (junction 7486) -> (junction 7489) [order 2]
                (junction 7487) -> (junction 7490) : {rpm1 = rpm1+100;}
                Flow.ButtonTriggeredMenu.Menu.MainSubMenu.SettingsSubMenu.ShiftLightSubMenu.RPM1menu.RPM1menu1 -> (junction 7488) [order 3] : SET || EXIT
                (junction 7488) -> (junction 7491) [order 2]
                (junction 7488) -> (junction 7492) [order 1] : [rpm1 > rpm2]
                (junction 7489) -> Flow.ButtonTriggeredMenu.Menu.MainSubMenu.SettingsSubMenu.ShiftLightSubMenu.RPM1menu.RPM1menu1
                (junction 7490) -> (junction 7489)
                (junction 7493) -> (junction 7489) [order 2]
                (junction 7494) -> (junction 7490) : {rpm1 = rpm1-100;}
                (junction 7492) -> (junction 7491) : {rpm2 = rpm1;}
                (junction 7491) -> 7496
                Flow.ButtonTriggeredMenu.Menu.MainSubMenu.SettingsSubMenu.ShiftLightSubMenu.RPM1menu.RPM1menu1 -> (junction 7493) [order 2] : DOWN
                (junction 7493) -> (junction 7494) [order 1] : [rpm1>5000 && rpm1 <= 11000]
              state RPM2menu
                state RPM1menu1
                  en:
                  currentState = eStateName.SL_SET_RPM2;
                (junction 7520) -> (junction 7521) [order 1] : [rpm2>=5000]
                7532 -> (junction 7520)
                (default) -> (junction 7520)
                (junction 7520) -> (junction 7522) [order 2]
                (junction 7521) -> Flow.ButtonTriggeredMenu.Menu.MainSubMenu.SettingsSubMenu.ShiftLightSubMenu.RPM2menu.RPM1menu1
                (junction 7522) -> Flow.ButtonTriggeredMenu.Menu.MainSubMenu.SettingsSubMenu.ShiftLightSubMenu.RPM2menu.RPM1menu1 : {rpm2 = 5000;}
                Flow.ButtonTriggeredMenu.Menu.MainSubMenu.SettingsSubMenu.ShiftLightSubMenu.RPM2menu.RPM1menu1 -> (junction 7523) [order 1] : UP
                (junction 7523) -> (junction 7524) [order 1] : [rpm2 >=5000 && rpm2<11000]
                (junction 7523) -> (junction 7527) [order 2]
                (junction 7524) -> (junction 7526) : {rpm2 = rpm2+100;}
                Flow.ButtonTriggeredMenu.Menu.MainSubMenu.SettingsSubMenu.ShiftLightSubMenu.RPM2menu.RPM1menu1 -> (junction 7525) [order 3] : SET || EXIT
                (junction 7525) -> (junction 7528) [order 2]
                (junction 7525) -> (junction 7529) [order 1] : [ rpm2 < rpm1]
                (junction 7526) -> (junction 7527)
                (junction 7527) -> Flow.ButtonTriggeredMenu.Menu.MainSubMenu.SettingsSubMenu.ShiftLightSubMenu.RPM2menu.RPM1menu1
                (junction 7531) -> (junction 7526) : {rpm2 = rpm2-100;}
                (junction 7530) -> (junction 7527) [order 2]
                (junction 7529) -> (junction 7528) : {rpm1 = rpm2;}
                (junction 7528) -> 7533
                Flow.ButtonTriggeredMenu.Menu.MainSubMenu.SettingsSubMenu.ShiftLightSubMenu.RPM2menu.RPM1menu1 -> (junction 7530) [order 2] : DOWN
                (junction 7530) -> (junction 7531) [order 1] : [rpm2 >5000 && rpm2<=11000]
              state ShiftLightSubMenu
                state ShiftLightOnOffMenu
                  (history)
                  state ShiftLightON
                    en:
                    currentState = eStateName.SHIFT_LIGHT_ON;
                  state ShiftLightOFF
                    en:
                    currentState = eStateName.SHIFT_LIGHT_OFF;
                  ShiftLightON -> ShiftLightOFF [order 1] : DOWN || UP
                  ShiftLightOFF -> ShiftLightON [order 1] : UP || DOWN
                7560 -> ShiftLightON
                ShiftLightON -> (junction 7557) [order 2] : SET
                (junction 7557) -> (junction 7558) : {BLU_FnShiftLightON();
                    | ShiftLightStatus = 1;}
                ShiftLightOnOffMenu -> (junction 7558) : EXIT
                (junction 7558) -> 7561
                (junction 7559) -> (junction 7558) : {BLU_FnShiftLightOFF();
                    | ShiftLightStatus = 0;}
                ShiftLightOFF -> (junction 7559) [order 2] : SET
              state OdoLessMiles
                en:
                currentState = eStateName.ODO_MSG_MILES;
              (junction 7422) -> (junction 7421)
              (junction 7421) -> 7436
              ShiftLightMenu1 -> (junction 7422) : EXIT
              SL_RPM1 -> 7432 [order 1] : SET
              SL_RPM1 -> (junction 7423) [order 3] : UP
              OdoLessKm -> (junction 7428) : [after(5,sec)]
              (junction 7425) -> OdoLessKm [order 2]
              (junction 7423) -> (junction 7426)
              7433 -> ShiftLightMenu1
              7434 -> (junction 7424)
              (junction 7424) -> (junction 7425) [order 1] : [odoUnits == 0]
              (junction 7425) -> (junction 7426) [order 1] : [odoValue > 1000]
              (junction 7426) -> (junction 7430)
              (junction 7424) -> (junction 7429) [order 2]
              (junction 7427) -> 7435 : [ShiftLightStatus == 1]
              SL_RPM2 -> (junction 7427) [order 1] : SET
              (junction 7428) -> 7436
              OdoLessMiles -> (junction 7428) : [after(5,sec)]
              7437 -> ShiftLightMenu1
              (junction 7429) -> (junction 7430) [order 1] : [odoValue > 600]
              (junction 7429) -> OdoLessMiles [order 2]
              (junction 7430) -> (junction 7431)
              (junction 7470) -> 7438 [order 1] : SET
              (junction 7431) -> Flow.ButtonTriggeredMenu.Menu.MainSubMenu.SettingsSubMenu.ShiftLightSubMenu.ShiftLightMenu1.ShiftLight
              7439 -> ShiftLightMenu1
            state ClockSubMenu
              (history)
              state ClockDateMenu
                (history)
                state Time
                  en:
                  currentState = eStateName.CLOCK_TIME;
                state Date
                  en:
                  currentState = eStateName.CLOCK_DATE;
                state ClockFormat
                  en:
                  currentState = eStateName.CLOCK_CLOCK_FORMAT;
                state DateFormat
                  en:
                  currentState = eStateName.CLOCK_DATE_FORMAT;
                (default) -> (junction 7612)
                (junction 7612) -> Time
                Time -> (junction 7613) [order 2]
                (junction 7615) -> (junction 7612)
                (junction 7613) -> (junction 7614) [order 2] : UP
                Time -> Date [order 1] : DOWN
                Date -> Time [order 1] : UP
                Date -> ClockFormat [order 2] : DOWN
                ClockFormat -> Date [order 2] : UP
                ClockFormat -> DateFormat [order 1] : DOWN
                DateFormat -> ClockFormat [order 1] : UP
                (junction 7614) -> DateFormat
                DateFormat -> (junction 7615) [order 2] : DOWN
              state TimeSubMenu
                (history)
                state TimeHours
                  en:
                  currentState = eStateName.TIME_HOURS;
                state TimeMinutes
                  en:
                  currentState = eStateName.TIME_MINUTES;
                TimeHours -> (junction 7634) [order 5] : longUP
                    | {jumpDigits = 5;}
                (junction 7634) -> (junction 7635)
                TimeHours -> (junction 7635) [order 3] : UP
                    | {jumpDigits=1;}
                (junction 7635) -> (junction 7636) : {BLU_FnClkIncHrs(jumpDigits);}
                (junction 7636) -> TimeHours
                (junction 7637) -> (junction 7636) : {BLU_FnClkDecHrs(jumpDigits);}
                7645 -> TimeHours
                TimeHours -> (junction 7637) [order 4] : DOWN
                    | {jumpDigits=1;}
                (junction 7638) -> (junction 7637)
                TimeHours -> (junction 7638) [order 6] : longDOWN
                    | {jumpDigits=5;}
                TimeHours -> (junction 7639) [order 2] : EXIT
                TimeHours -> TimeMinutes [order 1] : SET
                TimeMinutes -> TimeHours [order 1] : EXIT
                (junction 7639) -> 7646
                TimeMinutes -> (junction 7639) [order 2] : SET
                    | {BLU_FnClkSaveTime();}
                TimeMinutes -> (junction 7640) [order 6] : longUP
                    | {jumpDigits=5;}
                (junction 7640) -> (junction 7641)
                TimeMinutes -> (junction 7641) [order 3] : UP
                    | {jumpDigits=1;}
                (junction 7641) -> (junction 7642) : {BLU_FnClkIncMins(jumpDigits);}
                (junction 7642) -> TimeMinutes
                (junction 7643) -> (junction 7642) : {BLU_FnClkDecMins(jumpDigits);}
                TimeMinutes -> (junction 7643) [order 4] : DOWN
                    | {jumpDigits=1;}
                (junction 7644) -> (junction 7643)
                TimeMinutes -> (junction 7644) [order 5] : longDOWN
                    | {jumpDigits=5;}
              state DateSubMenu
                (history)
                state DateDDMMYYYY
                  (history)
                  state DateDigit1
                    en:
                    currentState = eStateName.CLOCK_FORMAT_DIGIT_1;
                  state DateDigit2
                    en:
                    currentState = eStateName.CLOCK_FORMAT_DIGIT_2;
                  state DateDigit3
                    en:
                    currentState = eStateName.CLOCK_FORMAT_DIGIT_3;
                  Flow.ButtonTriggeredMenu.Menu.MainSubMenu.SettingsSubMenu.ClockSubMenu.DateSubMenu.DateDDMMYYYY.DateDigit1 -> (junction 7699) [order 3] : longUP
                      | {BLU_FnClkIncDateDig1();}
                  (junction 7699) -> (junction 7700)
                  Flow.ButtonTriggeredMenu.Menu.MainSubMenu.SettingsSubMenu.ClockSubMenu.DateSubMenu.DateDDMMYYYY.DateDigit1 -> (junction 7700) [order 1] : UP
                      | {BLU_FnClkIncDateDig1();}
                  7717 -> Flow.ButtonTriggeredMenu.Menu.MainSubMenu.SettingsSubMenu.ClockSubMenu.DateSubMenu.DateDDMMYYYY.DateDigit1
                  (junction 7700) -> (junction 7702)
                  Flow.ButtonTriggeredMenu.Menu.MainSubMenu.SettingsSubMenu.ClockSubMenu.DateSubMenu.DateDDMMYYYY.DateDigit1 -> (junction 7701) [order 5] : EXIT
                  Flow.ButtonTriggeredMenu.Menu.MainSubMenu.SettingsSubMenu.ClockSubMenu.DateSubMenu.DateDDMMYYYY.DateDigit1 -> (junction 7702) [order 2] : DOWN
                      | {BLU_FnClkDecDateDig1();}
                  (junction 7701) -> (junction 7710)
                  (junction 7702) -> (junction 7703)
                  Flow.ButtonTriggeredMenu.Menu.MainSubMenu.SettingsSubMenu.ClockSubMenu.DateSubMenu.DateDDMMYYYY.DateDigit1 -> (junction 7703) [order 6] : longDOWN
                      | {BLU_FnClkDecDateDig1();}
                  (junction 7703) -> (junction 7704)
                  (junction 7704) -> Flow.ButtonTriggeredMenu.Menu.MainSubMenu.SettingsSubMenu.ClockSubMenu.DateSubMenu.DateDDMMYYYY.DateDigit1
                  Flow.ButtonTriggeredMenu.Menu.MainSubMenu.SettingsSubMenu.ClockSubMenu.DateSubMenu.DateDDMMYYYY.DateDigit1 -> Flow.ButtonTriggeredMenu.Menu.MainSubMenu.SettingsSubMenu.ClockSubMenu.DateSubMenu.DateDDMMYYYY.DateDigit2 [order 4] : SET
                  Flow.ButtonTriggeredMenu.Menu.MainSubMenu.SettingsSubMenu.ClockSubMenu.DateSubMenu.DateDDMMYYYY.DateDigit2 -> Flow.ButtonTriggeredMenu.Menu.MainSubMenu.SettingsSubMenu.ClockSubMenu.DateSubMenu.DateDDMMYYYY.DateDigit1 [order 1] : EXIT
                  Flow.ButtonTriggeredMenu.Menu.MainSubMenu.SettingsSubMenu.ClockSubMenu.DateSubMenu.DateDDMMYYYY.DateDigit2 -> (junction 7705) [order 2] : UP
                      | {BLU_FnClkIncDateDig2();}
                  (junction 7705) -> (junction 7706)
                  Flow.ButtonTriggeredMenu.Menu.MainSubMenu.SettingsSubMenu.ClockSubMenu.DateSubMenu.DateDDMMYYYY.DateDigit2 -> (junction 7706) [order 5] : longUP
                      | {BLU_FnClkIncDateDig2();}
                  (junction 7706) -> (junction 7707)
                  Flow.ButtonTriggeredMenu.Menu.MainSubMenu.SettingsSubMenu.ClockSubMenu.DateSubMenu.DateDDMMYYYY.DateDigit2 -> (junction 7707) [order 3] : DOWN
                      | {BLU_FnClkDecDateDig2();}
                  (junction 7707) -> (junction 7708)
                  Flow.ButtonTriggeredMenu.Menu.MainSubMenu.SettingsSubMenu.ClockSubMenu.DateSubMenu.DateDDMMYYYY.DateDigit2 -> (junction 7708) [order 6] : longDOWN
                      | {BLU_FnClkDecDateDig2();}
                  (junction 7708) -> (junction 7709)
                  (junction 7709) -> Flow.ButtonTriggeredMenu.Menu.MainSubMenu.SettingsSubMenu.ClockSubMenu.DateSubMenu.DateDDMMYYYY.DateDigit2
                  (junction 7710) -> 7718
                  (junction 7713) -> (junction 7710) : {BLU_FnClkSaveDate();}
                  Flow.ButtonTriggeredMenu.Menu.MainSubMenu.SettingsSubMenu.ClockSubMenu.DateSubMenu.DateDDMMYYYY.DateDigit2 -> Flow.ButtonTriggeredMenu.Menu.MainSubMenu.SettingsSubMenu.ClockSubMenu.DateSubMenu.DateDDMMYYYY.DateDigit3 [order 4] : SET
                  Flow.ButtonTriggeredMenu.Menu.MainSubMenu.SettingsSubMenu.ClockSubMenu.DateSubMenu.DateDDMMYYYY.DateDigit3 -> Flow.ButtonTriggeredMenu.Menu.MainSubMenu.SettingsSubMenu.ClockSubMenu.DateSubMenu.DateDDMMYYYY.DateDigit2 [order 3] : EXIT
                  Flow.ButtonTriggeredMenu.Menu.MainSubMenu.SettingsSubMenu.ClockSubMenu.DateSubMenu.DateDDMMYYYY.DateDigit3 -> (junction 7711) [order 1] : UP
                      | {BLU_FnClkIncDateDig3();}
                  (junction 7711) -> (junction 7712)
                  Flow.ButtonTriggeredMenu.Menu.MainSubMenu.SettingsSubMenu.ClockSubMenu.DateSubMenu.DateDDMMYYYY.DateDigit3 -> (junction 7712) [order 5] : longUP
                      | {BLU_FnClkIncDateDig3();}
                  (junction 7712) -> (junction 7714)
                  Flow.ButtonTriggeredMenu.Menu.MainSubMenu.SettingsSubMenu.ClockSubMenu.DateSubMenu.DateDDMMYYYY.DateDigit3 -> (junction 7713) [order 4] : SET
                  Flow.ButtonTriggeredMenu.Menu.MainSubMenu.SettingsSubMenu.ClockSubMenu.DateSubMenu.DateDDMMYYYY.DateDigit3 -> (junction 7714) [order 2] : DOWN
                      | {BLU_FnClkDecDateDig3();}
                  (junction 7714) -> (junction 7715)
                  Flow.ButtonTriggeredMenu.Menu.MainSubMenu.SettingsSubMenu.ClockSubMenu.DateSubMenu.DateDDMMYYYY.DateDigit3 -> (junction 7715) [order 6] : longDOWN
                      | {BLU_FnClkDecDateDig3();}
                  (junction 7715) -> (junction 7716)
                  (junction 7716) -> Flow.ButtonTriggeredMenu.Menu.MainSubMenu.SettingsSubMenu.ClockSubMenu.DateSubMenu.DateDDMMYYYY.DateDigit3
                state DateMMDDYYYY
                  (history)
                  state DateDigit1
                    en:
                    currentState = eStateName.CLOCK_FORMAT_DIGIT_2;
                  state DateDigit2
                    en:
                    currentState = eStateName.CLOCK_FORMAT_DIGIT_1;
                  state DateDigit3
                    en:
                    currentState = eStateName.CLOCK_FORMAT_DIGIT_3;
                  Flow.ButtonTriggeredMenu.Menu.MainSubMenu.SettingsSubMenu.ClockSubMenu.DateSubMenu.DateMMDDYYYY.DateDigit1 -> (junction 7760) [order 3] : UP
                      | {BLU_FnClkIncDateDig2();}
                  7778 -> Flow.ButtonTriggeredMenu.Menu.MainSubMenu.SettingsSubMenu.ClockSubMenu.DateSubMenu.DateMMDDYYYY.DateDigit1
                  (junction 7760) -> (junction 7762)
                  Flow.ButtonTriggeredMenu.Menu.MainSubMenu.SettingsSubMenu.ClockSubMenu.DateSubMenu.DateMMDDYYYY.DateDigit1 -> (junction 7761) [order 2] : EXIT
                  Flow.ButtonTriggeredMenu.Menu.MainSubMenu.SettingsSubMenu.ClockSubMenu.DateSubMenu.DateMMDDYYYY.DateDigit1 -> (junction 7762) [order 4] : longUP
                      | {BLU_FnClkIncDateDig2();}
                  (junction 7761) -> (junction 7771)
                  (junction 7762) -> (junction 7763)
                  Flow.ButtonTriggeredMenu.Menu.MainSubMenu.SettingsSubMenu.ClockSubMenu.DateSubMenu.DateMMDDYYYY.DateDigit1 -> (junction 7763) [order 5] : DOWN
                      | {BLU_FnClkDecDateDig2();}
                  (junction 7763) -> (junction 7764)
                  Flow.ButtonTriggeredMenu.Menu.MainSubMenu.SettingsSubMenu.ClockSubMenu.DateSubMenu.DateMMDDYYYY.DateDigit1 -> (junction 7764) [order 6] : longDOWN
                      | {BLU_FnClkDecDateDig2();}
                  (junction 7764) -> (junction 7765)
                  (junction 7765) -> Flow.ButtonTriggeredMenu.Menu.MainSubMenu.SettingsSubMenu.ClockSubMenu.DateSubMenu.DateMMDDYYYY.DateDigit1
                  Flow.ButtonTriggeredMenu.Menu.MainSubMenu.SettingsSubMenu.ClockSubMenu.DateSubMenu.DateMMDDYYYY.DateDigit1 -> Flow.ButtonTriggeredMenu.Menu.MainSubMenu.SettingsSubMenu.ClockSubMenu.DateSubMenu.DateMMDDYYYY.DateDigit2 [order 1] : SET
                  Flow.ButtonTriggeredMenu.Menu.MainSubMenu.SettingsSubMenu.ClockSubMenu.DateSubMenu.DateMMDDYYYY.DateDigit2 -> Flow.ButtonTriggeredMenu.Menu.MainSubMenu.SettingsSubMenu.ClockSubMenu.DateSubMenu.DateMMDDYYYY.DateDigit1 [order 1] : EXIT
                  Flow.ButtonTriggeredMenu.Menu.MainSubMenu.SettingsSubMenu.ClockSubMenu.DateSubMenu.DateMMDDYYYY.DateDigit2 -> (junction 7766) [order 6] : longUP
                      | {BLU_FnClkIncDateDig1();}
                  (junction 7766) -> (junction 7767)
                  Flow.ButtonTriggeredMenu.Menu.MainSubMenu.SettingsSubMenu.ClockSubMenu.DateSubMenu.DateMMDDYYYY.DateDigit2 -> (junction 7767) [order 2] : UP
                      | {BLU_FnClkIncDateDig1();}
                  (junction 7767) -> (junction 7768)
                  Flow.ButtonTriggeredMenu.Menu.MainSubMenu.SettingsSubMenu.ClockSubMenu.DateSubMenu.DateMMDDYYYY.DateDigit2 -> (junction 7768) [order 3] : DOWN
                      | {BLU_FnClkDecDateDig1();}
                  (junction 7768) -> (junction 7769)
                  Flow.ButtonTriggeredMenu.Menu.MainSubMenu.SettingsSubMenu.ClockSubMenu.DateSubMenu.DateMMDDYYYY.DateDigit2 -> (junction 7769) [order 4] : longDOWN
                      | {BLU_FnClkDecDateDig1();}
                  (junction 7769) -> (junction 7770)
                  (junction 7770) -> Flow.ButtonTriggeredMenu.Menu.MainSubMenu.SettingsSubMenu.ClockSubMenu.DateSubMenu.DateMMDDYYYY.DateDigit2
                  Flow.ButtonTriggeredMenu.Menu.MainSubMenu.SettingsSubMenu.ClockSubMenu.DateSubMenu.DateMMDDYYYY.DateDigit2 -> Flow.ButtonTriggeredMenu.Menu.MainSubMenu.SettingsSubMenu.ClockSubMenu.DateSubMenu.DateMMDDYYYY.DateDigit3 [order 5] : SET
                  Flow.ButtonTriggeredMenu.Menu.MainSubMenu.SettingsSubMenu.ClockSubMenu.DateSubMenu.DateMMDDYYYY.DateDigit3 -> Flow.ButtonTriggeredMenu.Menu.MainSubMenu.SettingsSubMenu.ClockSubMenu.DateSubMenu.DateMMDDYYYY.DateDigit2 [order 1] : EXIT
                  (junction 7771) -> 7779
                  (junction 7775) -> (junction 7771) : {BLU_FnClkSaveDate();}
                  Flow.ButtonTriggeredMenu.Menu.MainSubMenu.SettingsSubMenu.ClockSubMenu.DateSubMenu.DateMMDDYYYY.DateDigit3 -> (junction 7772) [order 4] : UP
                      | {BLU_FnClkIncDateDig3();}
                  (junction 7772) -> (junction 7773)
                  Flow.ButtonTriggeredMenu.Menu.MainSubMenu.SettingsSubMenu.ClockSubMenu.DateSubMenu.DateMMDDYYYY.DateDigit3 -> (junction 7773) [order 5] : longUP
                      | {BLU_FnClkIncDateDig3();}
                  (junction 7773) -> (junction 7774)
                  Flow.ButtonTriggeredMenu.Menu.MainSubMenu.SettingsSubMenu.ClockSubMenu.DateSubMenu.DateMMDDYYYY.DateDigit3 -> (junction 7774) [order 3] : DOWN
                      | {BLU_FnClkDecDateDig3();}
                  (junction 7774) -> (junction 7776)
                  Flow.ButtonTriggeredMenu.Menu.MainSubMenu.SettingsSubMenu.ClockSubMenu.DateSubMenu.DateMMDDYYYY.DateDigit3 -> (junction 7775) [order 6] : SET
                  Flow.ButtonTriggeredMenu.Menu.MainSubMenu.SettingsSubMenu.ClockSubMenu.DateSubMenu.DateMMDDYYYY.DateDigit3 -> (junction 7776) [order 2] : longDOWN
                      | {BLU_FnClkDecDateDig3();}
                  (junction 7776) -> (junction 7777)
                  (junction 7777) -> Flow.ButtonTriggeredMenu.Menu.MainSubMenu.SettingsSubMenu.ClockSubMenu.DateSubMenu.DateMMDDYYYY.DateDigit3
                state DateYYYYMMDD
                  (history)
                  state DateDigit1
                    en:
                    currentState = eStateName.CLOCK_FORMAT_DIGIT_3;
                  state DateDigit2
                    en:
                    currentState = eStateName.CLOCK_FORMAT_DIGIT_2;
                  state DateDigit3
                    en:
                    currentState = eStateName.CLOCK_FORMAT_DIGIT_1;
                  Flow.ButtonTriggeredMenu.Menu.MainSubMenu.SettingsSubMenu.ClockSubMenu.DateSubMenu.DateYYYYMMDD.DateDigit1 -> (junction 7821) [order 4] : UP
                      | {BLU_FnClkIncDateDig3();}
                  (junction 7821) -> (junction 7823)
                  7839 -> Flow.ButtonTriggeredMenu.Menu.MainSubMenu.SettingsSubMenu.ClockSubMenu.DateSubMenu.DateYYYYMMDD.DateDigit1
                  Flow.ButtonTriggeredMenu.Menu.MainSubMenu.SettingsSubMenu.ClockSubMenu.DateSubMenu.DateYYYYMMDD.DateDigit1 -> (junction 7822) [order 3] : EXIT
                  Flow.ButtonTriggeredMenu.Menu.MainSubMenu.SettingsSubMenu.ClockSubMenu.DateSubMenu.DateYYYYMMDD.DateDigit1 -> (junction 7823) [order 5] : longUP
                      | {BLU_FnClkIncDateDig3();}
                  (junction 7822) -> (junction 7832)
                  (junction 7823) -> (junction 7824)
                  Flow.ButtonTriggeredMenu.Menu.MainSubMenu.SettingsSubMenu.ClockSubMenu.DateSubMenu.DateYYYYMMDD.DateDigit1 -> (junction 7824) [order 6] : DOWN
                      | {BLU_FnClkDecDateDig3();}
                  (junction 7824) -> (junction 7825)
                  Flow.ButtonTriggeredMenu.Menu.MainSubMenu.SettingsSubMenu.ClockSubMenu.DateSubMenu.DateYYYYMMDD.DateDigit1 -> (junction 7825) [order 2] : longDOWN
                      | {BLU_FnClkDecDateDig3();}
                  (junction 7825) -> (junction 7826)
                  (junction 7826) -> Flow.ButtonTriggeredMenu.Menu.MainSubMenu.SettingsSubMenu.ClockSubMenu.DateSubMenu.DateYYYYMMDD.DateDigit1
                  Flow.ButtonTriggeredMenu.Menu.MainSubMenu.SettingsSubMenu.ClockSubMenu.DateSubMenu.DateYYYYMMDD.DateDigit1 -> Flow.ButtonTriggeredMenu.Menu.MainSubMenu.SettingsSubMenu.ClockSubMenu.DateSubMenu.DateYYYYMMDD.DateDigit2 [order 1] : SET
                  Flow.ButtonTriggeredMenu.Menu.MainSubMenu.SettingsSubMenu.ClockSubMenu.DateSubMenu.DateYYYYMMDD.DateDigit2 -> Flow.ButtonTriggeredMenu.Menu.MainSubMenu.SettingsSubMenu.ClockSubMenu.DateSubMenu.DateYYYYMMDD.DateDigit1 [order 1] : EXIT
                  Flow.ButtonTriggeredMenu.Menu.MainSubMenu.SettingsSubMenu.ClockSubMenu.DateSubMenu.DateYYYYMMDD.DateDigit2 -> (junction 7827) [order 2] : UP
                      | {BLU_FnClkIncDateDig2();}
                  (junction 7827) -> (junction 7828)
                  Flow.ButtonTriggeredMenu.Menu.MainSubMenu.SettingsSubMenu.ClockSubMenu.DateSubMenu.DateYYYYMMDD.DateDigit2 -> (junction 7828) [order 3] : longUP
                      | {BLU_FnClkIncDateDig2();}
                  (junction 7828) -> (junction 7829)
                  Flow.ButtonTriggeredMenu.Menu.MainSubMenu.SettingsSubMenu.ClockSubMenu.DateSubMenu.DateYYYYMMDD.DateDigit2 -> (junction 7829) [order 4] : DOWN
                      | {BLU_FnClkDecDateDig2();}
                  (junction 7829) -> (junction 7830)
                  Flow.ButtonTriggeredMenu.Menu.MainSubMenu.SettingsSubMenu.ClockSubMenu.DateSubMenu.DateYYYYMMDD.DateDigit2 -> (junction 7830) [order 5] : longDOWN
                      | {BLU_FnClkDecDateDig2();}
                  (junction 7830) -> (junction 7831)
                  (junction 7831) -> Flow.ButtonTriggeredMenu.Menu.MainSubMenu.SettingsSubMenu.ClockSubMenu.DateSubMenu.DateYYYYMMDD.DateDigit2
                  Flow.ButtonTriggeredMenu.Menu.MainSubMenu.SettingsSubMenu.ClockSubMenu.DateSubMenu.DateYYYYMMDD.DateDigit2 -> Flow.ButtonTriggeredMenu.Menu.MainSubMenu.SettingsSubMenu.ClockSubMenu.DateSubMenu.DateYYYYMMDD.DateDigit3 [order 6] : SET